simulink library "lib_all"  (Simulink 7.6)
settings: Solver=ode45  SolverMode=SingleTasking  StartTime=0.0  StopTime=10.0  RelTol=1e-3
values from neighbouring files (symbols this model uses):
  map_lib_03 = [6.8679 7.0159 7.4571 8.1849 9.1892 10.456 11.97 13.71 15.655 17.781 20.063 22.474 ... (803 elements, 73x11)]    [scr_simdata.mat]    (also in scr_simdata_all.mat)
  map_lib_06 = [2.5784 2.6727 2.9588 3.4453 4.1444 5.068 6.226 7.6242 9.2634 11.139 13.24 15.552 ... (803 elements, 73x11)]    [scr_simdata.mat]    (also in scr_simdata_all.mat)
  map_lib_09 = [0.0025784 0.0026727 0.0029588 0.0034453 0.0041444 0.005068 0.006226 0.0076242 0.0092634 0.011139 0.01324 0.015552 ... (803 elements, 73x11)]    [scr_simdata.mat]    (also in scr_simdata_all.mat)
  map_lib_10 = [2.74 2.75 2.76 2.77 2.78 2.79 2.8 2.81 2.82 2.83 2.84 2.85 ... (81 elements, 1x81)]    [scr_simdata.mat]    (also in scr_simdata_all.mat)
  map_lib_11 = [1.1983 1.2027 1.207 1.2113 1.2156 1.2198 1.2241 1.2283 1.2326 1.2368 1.241 1.2451 ... (81 elements, 1x81)]    [scr_simdata.mat]    (also in scr_simdata_all.mat)
  map_lib_12 = [2.74 2.75 2.76 2.77 2.78 2.79 2.8 2.81 2.82 2.83 2.84 2.85 ... (81 elements, 1x81)]    [scr_simdata.mat]    (also in scr_simdata_all.mat)
  map_lib_13 = [1.74 1.75 1.76 1.77 1.78 1.79 1.8 1.81 1.82 1.83 1.84 1.85 ... (81 elements, 1x81)]    [scr_simdata.mat]    (also in scr_simdata_all.mat)
  map_lib_16 = [0.00048828125 1.6159 1.8962 2.0821 2.2251 2.3426 2.4433 2.5318 2.611 2.683 2.749 2.8101 ... (1419 elements, 129x11)]    [scr_simdata.mat]    (also in scr_simdata_all.mat)
  map_mr2_01 = [0 800 1600 2400 3200 4000]    [scr_simdata.mat]    (also in scr_simdata_all.mat)
  map_mr2_02 = [1.32 1.32 1.33 1.34 1.36 1.38]    [scr_simdata.mat]    (also in scr_simdata_all.mat)
  map_mr2_03 = [0 800 1600 2400 3200 4000]    [scr_simdata.mat]    (also in scr_simdata_all.mat)
  map_mr2_04 = [1.32 1.32 1.33 1.34 1.36 1.38]    [scr_simdata.mat]    (also in scr_simdata_all.mat)
  map_mr2_05 = [0 800 1600 2400 3200 4000]    [scr_simdata.mat]    (also in scr_simdata_all.mat)
  map_mr2_06 = [1.32 1.32 1.33 1.34 1.36 1.38]    [scr_simdata.mat]    (also in scr_simdata_all.mat)
  Parameter1 = 6    [scr_simdata.mat]    (also in scr_simdata_all.mat)
  Parameter101 = 30    [scr_simdata.mat]    (also in scr_simdata_all.mat)
  Parameter102 = 12    [scr_simdata.mat]    (also in scr_simdata_all.mat)
  Parameter103 = -240    [scr_simdata.mat]    (also in scr_simdata_all.mat)
  Parameter108 = 48    [scr_simdata.mat]    (also in scr_simdata_all.mat)
  Parameter120 = 16    [scr_simdata.mat]    (also in scr_simdata_all.mat)
  Parameter121 = 87    [scr_simdata.mat]    (also in scr_simdata_all.mat)
  Parameter123 = 78    [scr_simdata.mat]    (also in scr_simdata_all.mat)
  Parameter124 = 60    [scr_simdata.mat]    (also in scr_simdata_all.mat)
  Parameter125 = -480    [scr_simdata.mat]    (also in scr_simdata_all.mat)
  Parameter130 = 96    [scr_simdata.mat]    (also in scr_simdata_all.mat)
  Parameter131 = 3    [scr_simdata.mat]    (also in scr_simdata_all.mat)
  Parameter144 = 3    [scr_simdata.mat]    (also in scr_simdata_all.mat)
  Parameter18 = 128    [scr_simdata.mat]    (also in scr_simdata_all.mat)
  Parameter19 = 16    [scr_simdata.mat]    (also in scr_simdata_all.mat)
  Parameter2 = 12    [scr_simdata.mat]    (also in scr_simdata_all.mat)
  Parameter20 = 7    [scr_simdata.mat]    (also in scr_simdata_all.mat)
  Parameter21 = 4    [scr_simdata.mat]    (also in scr_simdata_all.mat)
  Parameter3 = 18    [scr_simdata.mat]    (also in scr_simdata_all.mat)
  Parameter32 = 1    [scr_simdata.mat]    (also in scr_simdata_all.mat)
  Parameter34 = 24    [scr_simdata.mat]    (also in scr_simdata_all.mat)
  Parameter4 = 138    [scr_simdata.mat]    (also in scr_simdata_all.mat)
  Parameter42 = 48    [scr_simdata.mat]    (also in scr_simdata_all.mat)
  Parameter5 = 135    [scr_simdata.mat]    (also in scr_simdata_all.mat)
  Parameter55 = 0    [scr_simdata.mat]    (also in scr_simdata_all.mat)
  Parameter56 = 0    [scr_simdata.mat]    (also in scr_simdata_all.mat)
  Parameter57 = 1    [scr_simdata.mat]    (also in scr_simdata_all.mat)
  Parameter58 = 1    [scr_simdata.mat]    (also in scr_simdata_all.mat)
  Parameter59 = 2    [scr_simdata.mat]    (also in scr_simdata_all.mat)
  Parameter6 = 132    [scr_simdata.mat]    (also in scr_simdata_all.mat)
  Parameter60 = 2    [scr_simdata.mat]    (also in scr_simdata_all.mat)
  Parameter63 = 144    [scr_simdata.mat]    (also in scr_simdata_all.mat)
  Parameter7 = 126    [scr_simdata.mat]    (also in scr_simdata_all.mat)
  Parameter76 = 64    [scr_simdata.mat]    (also in scr_simdata_all.mat)
  Parameter77 = 135    [scr_simdata.mat]    (also in scr_simdata_all.mat)
  Parameter79 = 126    [scr_simdata.mat]    (also in scr_simdata_all.mat)
  Parameter80 = 108    [scr_simdata.mat]    (also in scr_simdata_all.mat)
  Parameter81 = 0    [scr_simdata.mat]    (also in scr_simdata_all.mat)
  Parameter86 = 0    [scr_simdata.mat]    (also in scr_simdata_all.mat)
  Parameter98 = 112    [scr_simdata.mat]    (also in scr_simdata_all.mat)
  Parameter99 = 39    [scr_simdata.mat]    (also in scr_simdata_all.mat)
undefined symbols (not defined in the file or in its folder): cylvolkp_hih_map2h, cylvolkp_map2h, pk1k_map2h
libraries: simulink x2
(part 10 of 36)

== system lib_all/SubSystem800/SubSystem955/SubSystem958 ==
  note: ***編集禁止***
  input : Inport
  element : Inport  Port=2
  Assignment : Assignment  IndexOptions=Index vector (port)  Indices=1  OutputSizes=[1 1]
  Inside_DSR : DataStoreRead  DataStoreName=DSM183
  Inside_DSW : DataStoreWrite  DataStoreName=DSM183
  wires:
  element/1 -> Assignment/3
  input/1 -> Assignment/2
  Inside_DSR/1 -> Assignment/1
  Assignment/1 -> Inside_DSW/1

== system lib_all/SubSystem959/SubSystem963/SubSystem1018 ==
  Block2612 : Inport
  Block2613 : Inport  Port=2
  Block2614 : Inport  Port=3
  Block2615 : Sum  OutDataTypeStr=double  RndMeth=Zero  SaturateOnIntegerOverflow=on  InputSameDT=on
  Block2616 : Sum  Inputs=+-  OutDataTypeStr=double  RndMeth=Zero  SaturateOnIntegerOverflow=on  InputSameDT=on
  Block2617 : Switch  Threshold=0.5  RndMeth=Zero  SaturateOnIntegerOverflow=on  InputSameDT=on
  Block2618 : Switch  Threshold=0.5  RndMeth=Zero  SaturateOnIntegerOverflow=on  InputSameDT=on
  SubSystem1019 : Subsystem
  SubSystem1020 : Subsystem
  Block2619 : Outport
  wires:
  Block2613/1 -> SubSystem1019/1(In1), Block2616/1, SubSystem1020/1(In1), Block2618/3, Block2615/2
  Block2612/1 -> Block2615/1, Block2616/2, SubSystem1019/2(In2)
  SubSystem1019/1(Out1) -> Block2617/2
  Block2614/1 -> SubSystem1020/2(In2)
  SubSystem1020/1(Out1) -> Block2618/2
  Block2618/1 -> Block2617/3
  Block2615/1 -> Block2618/1
  Block2617/1 -> Block2619/1
  Block2616/1 -> Block2617/1

== system lib_all/SubSystem959/SubSystem963/SubSystem1021 ==
  note: ***編集禁止***
  element : Inport
  Inside_DSR : DataStoreRead  DataStoreName=DSM49
  Selector : Selector  IndexOptions=Index vector (port)  Indices=[1 3]  OutputSizes=1
  Output : Outport
  wires:
  Selector/1 -> Output/1
  element/1 -> Selector/2
  Inside_DSR/1 -> Selector/1

== system lib_all/SubSystem959/SubSystem963/SubSystem1022 ==
  note: ***編集禁止***
  element : Inport
  Inside_DSR : DataStoreRead  DataStoreName=DSM36
  Selector : Selector  IndexOptions=Index vector (port)  Indices=[1 3]  OutputSizes=1
  Output : Outport
  wires:
  Selector/1 -> Output/1
  element/1 -> Selector/2
  Inside_DSR/1 -> Selector/1

== system lib_all/SubSystem959/SubSystem963/SubSystem964 ==
  note: ***編集禁止***
  In1 : Inport
  In2 : Inport  Port=2
  RO1 : RelationalOperator  Operator===  InputSameDT=on
  single1 : DataTypeConversion  OutDataTypeStr=single  SaturateOnIntegerOverflow=on  RndMeth=Zero
  single2 : DataTypeConversion  OutDataTypeStr=single  SaturateOnIntegerOverflow=on  RndMeth=Zero
  Out1 : Outport
  wires:
  RO1/1 -> Out1/1
  In2/1 -> single2/1
  In1/1 -> single1/1
  single1/1 -> RO1/1
  single2/1 -> RO1/2

== system lib_all/SubSystem959/SubSystem963/SubSystem965 ==
  note: Text185
  Block2490 : Inport
  Block2491 : Inport  Port=2
  Block2492 : Inport  Port=3
  Block2493 : Inport  Port=4
  Block2494 : Constant  Value=0  OutDataTypeStr=double  SampleTime=-1
  Block2495 : Constant  OutDataTypeStr=double  SampleTime=-1
  Block2496 : Constant  Value=0  OutDataTypeStr=double  SampleTime=-1
  Block2497 : Gain  Gain=1/5  SaturateOnIntegerOverflow=on  OutDataTypeStr=Inherit: Same as input  ParamDataTypeStr=Inherit: Same as input
      settings: AttributesFormatString=Attribute508
  Block2498 : Product  OutDataTypeStr=double  SaturateOnIntegerOverflow=on  RndMeth=Zero  InputSameDT=on
  Block2499 : Sum  Inputs=-+  OutDataTypeStr=double  RndMeth=Zero  SaturateOnIntegerOverflow=on  InputSameDT=on
  Block2500 : Sum  OutDataTypeStr=double  RndMeth=Zero  SaturateOnIntegerOverflow=on  InputSameDT=on
  Block2501 : Sum  OutDataTypeStr=double  RndMeth=Zero  SaturateOnIntegerOverflow=on  InputSameDT=on
  Block2502 : Switch  Threshold=0.5  RndMeth=Zero  SaturateOnIntegerOverflow=on  InputSameDT=on
  SubSystem967 : Subsystem
  SubSystem968 : Subsystem
  SubSystem974 : Subsystem
  Block2611 : Outport
  wires:
  Block2491/1 -> SubSystem968/1(Block2503)
  SubSystem968/1(Block2528) -> SubSystem974/1(Block2530)
  Block2493/1 -> SubSystem974/2(Block2531)
  SubSystem974/1(Block2609) -> Block2500/1, Block2499/1
  SubSystem974/2(Block2610) -> Block2499/2
  Block2499/1 -> Block2498/1  "SigLabel85"
  SubSystem968/2(Block2529) -> Block2498/2
  Block2500/1 -> Block2502/3, SubSystem967/1(In1)
  Block2492/1 -> SubSystem968/3(Block2505)
  Block2496/1 -> SubSystem967/2(In2)
  SubSystem967/1(Out1) -> Block2502/2
  Block2502/1 -> Block2611/1
  Block2494/1 -> Block2502/1
  Block2495/1 -> Block2501/2
  Block2498/1 -> Block2497/1
  Block2497/1 -> Block2500/2
  Block2490/1 -> Block2501/1
  Block2501/1 -> SubSystem968/2(Block2504), SubSystem974/3(Block2532)

== system lib_all/SubSystem1023/SubSystem1025/SubSystem1038/SubSystem1040 ==
  note: ***編集禁止***
  In1 : Inport
  In2 : Inport  Port=2
  RO1 : RelationalOperator  Operator===  InputSameDT=on
  single1 : DataTypeConversion  OutDataTypeStr=single  SaturateOnIntegerOverflow=on  RndMeth=Zero
  single2 : DataTypeConversion  OutDataTypeStr=single  SaturateOnIntegerOverflow=on  RndMeth=Zero
  Out1 : Outport
  wires:
  single2/1 -> RO1/2
  single1/1 -> RO1/1
  In1/1 -> single1/1
  In2/1 -> single2/1
  RO1/1 -> Out1/1

== system lib_all/SubSystem1023/SubSystem1025/SubSystem1038/SubSystem1041 ==
  note: Text189
  Block2707 : Inport
  Block2708 : Inport  Port=2
  Block2709 : Constant  Value=0  OutDataTypeStr=double  SampleTime=-1
  Block2710 : Constant  Value=720  OutDataTypeStr=double  SampleTime=-1
  Block2711 : Gain  Gain=5  SaturateOnIntegerOverflow=on  OutDataTypeStr=Inherit: Same as input  ParamDataTypeStr=Inherit: Same as input
      settings: AttributesFormatString=Attribute537
  SubSystem1042 : Subsystem
      settings: RTWFcnNameOpts=Use subsystem name
  SubSystem1047 : Subsystem
      settings: RTWSystemCode=Inline
  Block2737 : Outport
  wires:
  Block2709/1 -> SubSystem1047/3(Block2733)
  Block2710/1 -> SubSystem1047/1(Block2731)
  SubSystem1047/1(Block2736) -> Block2737/1
  Block2707/1 -> SubSystem1042/1(Block2712)
  Block2708/1 -> SubSystem1042/2(Block2713)
  SubSystem1042/1(Block2730) -> Block2711/1
  Block2711/1 -> SubSystem1047/2(Block2732)

== system lib_all/SubSystem1023/SubSystem1025/SubSystem1038/SubSystem1049 ==
  Block2738 : Inport
  Block2739 : Inport  Port=2
  Block2740 : Inport  Port=3
  Block2741 : EnablePort
  Block2742 : DataStoreWrite  DataStoreName=DSM12
  Block2743 : DataStoreWrite  DataStoreName=DSM14
  Block2744 : DataStoreWrite  DataStoreName=DSM13
  wires:
  Block2739/1 -> Block2743/1
  Block2740/1 -> Block2744/1
  Block2738/1 -> Block2742/1

== system lib_all/SubSystem1023/SubSystem1025/SubSystem1038/SubSystem1050 ==
  note: Text190
  Block2745 : Inport
  Block2746 : Inport  Port=2
  Block2747 : Constant  Value=360  OutDataTypeStr=double  SampleTime=-1
  Block2748 : Constant  Value=360  OutDataTypeStr=double  SampleTime=-1
  Block2749 : Sum  Inputs=+-  OutDataTypeStr=double  RndMeth=Zero  SaturateOnIntegerOverflow=on  InputSameDT=on
  Block2750 : Switch  Threshold=0.5  RndMeth=Zero  SaturateOnIntegerOverflow=on  InputSameDT=on
  Block2751 : Lookup2D  RowIndex=map_lib_05  ColumnIndex=map_lib_04  Table=map_lib_06  SaturateOnIntegerOverflow=on  InputSameDT=on
      settings: AttributesFormatString=<%<OutputValues>>\nAttribute546
  SubSystem1051 : Subsystem
  Block2752 : Outport
  wires:
  Block2751/1 -> Block2752/1
  Block2747/1 -> Block2749/2
  Block2749/1 -> Block2750/1
  SubSystem1051/1(Out1) -> Block2750/2
  Block2748/1 -> SubSystem1051/2(In2)
  Block2746/1 -> Block2749/1, Block2750/3, SubSystem1051/1(In1)
  Block2745/1 -> Block2751/2
  Block2750/1 -> Block2751/1  "SigLabel86"

== system lib_all/SubSystem1023/SubSystem1025/SubSystem1052/SubSystem1054 ==
  note: Text194
  note: Text193
  Block2763 : Inport
  Block2764 : Inport  Port=2
  Block2765 : Constant  Value=0  OutDataTypeStr=double  SampleTime=-1
  Block2766 : Constant  Value=0  OutDataTypeStr=double  SampleTime=-1
  Block2767 : Constant  Value=720  OutDataTypeStr=double  SampleTime=-1
  Block2768 : Constant  Value=5  OutDataTypeStr=double  SampleTime=-1
  Block2769 : DataStoreRead  DataStoreName=DSM50
  Block2770 : DataStoreRead  DataStoreName=DSM35
  Block2771 : Gain  Gain=5  SaturateOnIntegerOverflow=on  OutDataTypeStr=Inherit: Same as input  ParamDataTypeStr=Inherit: Same as input
      settings: AttributesFormatString=Attribute554
  Block2772 : Gain  Gain=5  SaturateOnIntegerOverflow=on  OutDataTypeStr=Inherit: Same as input  ParamDataTypeStr=Inherit: Same as input
      settings: AttributesFormatString=Attribute555
  Block2773 : Gain  Gain=1/5  SaturateOnIntegerOverflow=on  OutDataTypeStr=Inherit: Same as input  ParamDataTypeStr=Inherit: Same as input
      settings: AttributesFormatString=Attribute556
  Block2774 : Product  Inputs=*/  OutDataTypeStr=double  SaturateOnIntegerOverflow=on  RndMeth=Zero  InputSameDT=on
  Block2775 : Rounding
  Block2776 : Rounding
  Block2777 : Sum  Inputs=+-+  OutDataTypeStr=double  RndMeth=Zero  SaturateOnIntegerOverflow=on  InputSameDT=on
  Block2778 : Sum  Inputs=-+  OutDataTypeStr=double  RndMeth=Zero  SaturateOnIntegerOverflow=on  InputSameDT=on
  SubSystem1055 : Subsystem  TreatAsAtomicUnit=on  mask(***ATC.DIVISION***){bd_version=1.01.A, r0=チェック有り, r1=, r2=, r3=, r4=, r5=, r6=, r7=, r8=, r9=, c0=, c1=, c2=, c3=, c4=, c5=, c6=, c7=, c8=, c9=}
      mask prompts: bd_version "Version"
      mask help: これはAutoCからのブロックです．編集禁止．
      settings: OpenFcn=warndlg('変更禁止!');
      mask Help: web(ui_blockhelpmng(gcb));
  SubSystem1056 : Subsystem  TreatAsAtomicUnit=on  mask(***ATC.DSRVEC***){bd_version=1.01.A, r0=DSM95, r1=-1, r2=, r3=, r4=, r5=, r6=, r7=, r8=, r9=, c0=, c1=, c2=, c3=, c4=, c5=, c6=, c7=, c8=, c9=}
      mask prompts: bd_version "Version"
      mask help: これはAutoCからのブロックです．編集禁止．
      settings: OpenFcn=warndlg('変更禁止!');
      mask Help: web(ui_blockhelpmng(gcb));
      mask Display: disp('ATC.DSRVEC')
  SubSystem1057 : Subsystem
      settings: RTWSystemCode=Inline
  Block2787 : Outport
  Block2788 : Outport  Port=2
  wires:
  SubSystem1055/1(Out) -> Block2777/1  "SigLabel88"
  Block2763/1 -> Block2772/1
  Block2771/1 -> Block2778/1
  Block2775/1 -> Block2771/1
  Block2774/1 -> Block2775/1
  SubSystem1057/1(Block2786) -> Block2778/2, Block2773/1, Block2774/1
  Block2766/1 -> SubSystem1057/3(Block2781)
  Block2777/1 -> SubSystem1057/2(Block2780)  "SigLabel87"
  Block2767/1 -> SubSystem1057/1(Block2779)
  Block2764/1 -> SubSystem1056/1(element)
  Block2778/1 -> Block2788/1
  Block2768/1 -> Block2774/2
  Block2765/1 -> SubSystem1055/3(init)
  SubSystem1056/1(Output) -> SubSystem1055/1(mult)
  Block2770/1 -> SubSystem1055/2(div)
  Block2769/1 -> Block2777/2
  Block2772/1 -> Block2777/3
  Block2773/1 -> Block2776/1
  Block2776/1 -> Block2787/1

== system lib_all/SubSystem1023/SubSystem1025/SubSystem1052/SubSystem1060 ==
  note: Text197
  note: Text196
  note: Text195
  Block2789 : Inport
  Block2790 : Inport  Port=2
  Block2791 : Inport  Port=3
  Block2792 : Constant  Value=0  OutDataTypeStr=double  SampleTime=-1
  Block2793 : Constant  OutDataTypeStr=double  SampleTime=-1
  Block2794 : Constant  OutDataTypeStr=double  SampleTime=-1
  Block2795 : Constant  Value=0  OutDataTypeStr=double  SampleTime=-1
  Block2796 : Constant  OutDataTypeStr=double  SampleTime=-1
  Block2797 : Constant  Value=143  OutDataTypeStr=double  SampleTime=-1
  Block2798 : Demux  Outputs=2
  Block2799 : Logic  Operator=NOT
  Block2800 : Mux  Inputs=2
  Block2801 : Mux  Inputs=2
  Block2802 : Sum  OutDataTypeStr=double  RndMeth=Zero  SaturateOnIntegerOverflow=on  InputSameDT=on
  Block2803 : Sum  OutDataTypeStr=double  RndMeth=Zero  SaturateOnIntegerOverflow=on  InputSameDT=on
  Block2804 : Switch  Threshold=0.5  RndMeth=Zero  SaturateOnIntegerOverflow=on  InputSameDT=on
  Block2805 : Switch  Threshold=0.5  RndMeth=Zero  SaturateOnIntegerOverflow=on  InputSameDT=on
  Block2806 : Switch  Threshold=0.5  RndMeth=Zero  SaturateOnIntegerOverflow=on  InputSameDT=on
  SubSystem1061 : Subsystem
  SubSystem1062 : Subsystem
  SubSystem1063 : Subsystem
  SubSystem1064 : Subsystem  TreatAsAtomicUnit=on
  SubSystem1073 : Subsystem  TreatAsAtomicUnit=on
  SubSystem1083 : Subsystem  TreatAsAtomicUnit=on
  Block2851 : Outport
  Block2852 : Outport  Port=2
  wires:
  Block2803/1 -> SubSystem1083/1(Block2836), SubSystem1073/1(Block2821), SubSystem1064/1(Block2807)
  Block2796/1 -> Block2803/1
  Block2806/1 -> Block2803/2
  Block2791/1 -> SubSystem1083/2(Block2837), SubSystem1073/2(Block2822), SubSystem1064/2(Block2808)
  Block2800/1 -> Block2806/1
  Block2802/1 -> Block2800/2
  Block2801/1 -> Block2806/3
  Block2795/1 -> Block2801/2
  Block2794/1 -> Block2802/1
  SubSystem1061/1(Out1) -> Block2806/2
  Block2804/1 -> Block2798/1
  Block2798/2 -> Block2852/1
  Block2798/1 -> Block2851/1
  SubSystem1083/1(Block2850) -> Block2805/3
  Block2805/1 -> Block2804/3
  Block2799/1 -> SubSystem1083/Enable
  SubSystem1073/1(Block2835) -> Block2805/1
  SubSystem1063/1(Out1) -> Block2799/1, SubSystem1073/Enable, Block2805/2
  Block2793/1 -> SubSystem1063/2(In2)
  SubSystem1064/1(Block2820) -> Block2804/1
  SubSystem1062/1(Out1) -> SubSystem1064/Enable, Block2804/2
  Block2792/1 -> SubSystem1062/2(In2)
  Block2790/1 -> SubSystem1063/1(In1), SubSystem1062/1(In1)
  Block2797/1 -> Block2801/1, SubSystem1061/2(In2)
  Block2789/1 -> Block2800/1, Block2802/2, SubSystem1061/1(In1)

== system lib_all/SubSystem1023/SubSystem1093/SubSystem1106/SubSystem1108 ==
  note: ***編集禁止***
  In1 : Inport
  In2 : Inport  Port=2
  RO1 : RelationalOperator  Operator===  InputSameDT=on
  single1 : DataTypeConversion  OutDataTypeStr=single  SaturateOnIntegerOverflow=on  RndMeth=Zero
  single2 : DataTypeConversion  OutDataTypeStr=single  SaturateOnIntegerOverflow=on  RndMeth=Zero
  Out1 : Outport
  wires:
  single2/1 -> RO1/2
  single1/1 -> RO1/1
  In1/1 -> single1/1
  In2/1 -> single2/1
  RO1/1 -> Out1/1

== system lib_all/SubSystem1023/SubSystem1093/SubSystem1106/SubSystem1109 ==
  note: Text208
  Block2932 : Inport
  Block2933 : Inport  Port=2
  Block2934 : Constant  Value=0  OutDataTypeStr=double  SampleTime=-1
  Block2935 : Constant  Value=720  OutDataTypeStr=double  SampleTime=-1
  Block2936 : Gain  Gain=5  SaturateOnIntegerOverflow=on  OutDataTypeStr=Inherit: Same as input  ParamDataTypeStr=Inherit: Same as input
      settings: AttributesFormatString=Attribute574
  SubSystem1110 : Subsystem
      settings: RTWFcnNameOpts=Use subsystem name
  SubSystem1115 : Subsystem
      settings: RTWSystemCode=Inline
  Block2962 : Outport
  wires:
  Block2934/1 -> SubSystem1115/3(Block2958)
  Block2935/1 -> SubSystem1115/1(Block2956)
  SubSystem1115/1(Block2961) -> Block2962/1
  Block2932/1 -> SubSystem1110/1(Block2937)
  Block2933/1 -> SubSystem1110/2(Block2938)
  SubSystem1110/1(Block2955) -> Block2936/1
  Block2936/1 -> SubSystem1115/2(Block2957)

== system lib_all/SubSystem1023/SubSystem1093/SubSystem1106/SubSystem1117 ==
  Block2963 : Inport
  Block2964 : Inport  Port=2
  Block2965 : Inport  Port=3
  Block2966 : EnablePort
  Block2967 : DataStoreWrite  DataStoreName=DSM12
  Block2968 : DataStoreWrite  DataStoreName=DSM14
  Block2969 : DataStoreWrite  DataStoreName=DSM13
  wires:
  Block2964/1 -> Block2968/1
  Block2965/1 -> Block2969/1
  Block2963/1 -> Block2967/1

== system lib_all/SubSystem1023/SubSystem1093/SubSystem1106/SubSystem1118 ==
  note: Text209
  Block2970 : Inport
  Block2971 : Inport  Port=2
  Block2972 : Constant  Value=360  OutDataTypeStr=double  SampleTime=-1
  Block2973 : Constant  Value=360  OutDataTypeStr=double  SampleTime=-1
  Block2974 : Sum  Inputs=+-  OutDataTypeStr=double  RndMeth=Zero  SaturateOnIntegerOverflow=on  InputSameDT=on
  Block2975 : Switch  Threshold=0.5  RndMeth=Zero  SaturateOnIntegerOverflow=on  InputSameDT=on
  Block2976 : Lookup2D  RowIndex=map_lib_05  ColumnIndex=map_lib_04  Table=map_lib_06  SaturateOnIntegerOverflow=on  InputSameDT=on
      settings: AttributesFormatString=<%<OutputValues>>\nAttribute583
  SubSystem1119 : Subsystem
  Block2977 : Outport
  wires:
  Block2976/1 -> Block2977/1
  Block2972/1 -> Block2974/2
  Block2974/1 -> Block2975/1
  SubSystem1119/1(Out1) -> Block2975/2
  Block2973/1 -> SubSystem1119/2(In2)
  Block2971/1 -> Block2974/1, Block2975/3, SubSystem1119/1(In1)
  Block2970/1 -> Block2976/2
  Block2975/1 -> Block2976/1  "SigLabel96"

== system lib_all/SubSystem1023/SubSystem1093/SubSystem1120/SubSystem1122 ==
  note: Text213
  note: Text212
  Block2988 : Inport
  Block2989 : Inport  Port=2
  Block2990 : Constant  Value=0  OutDataTypeStr=double  SampleTime=-1
  Block2991 : Constant  Value=0  OutDataTypeStr=double  SampleTime=-1
  Block2992 : Constant  Value=720  OutDataTypeStr=double  SampleTime=-1
  Block2993 : Constant  Value=5  OutDataTypeStr=double  SampleTime=-1
  Block2994 : DataStoreRead  DataStoreName=DSM50
  Block2995 : DataStoreRead  DataStoreName=DSM35
  Block2996 : Gain  Gain=5  SaturateOnIntegerOverflow=on  OutDataTypeStr=Inherit: Same as input  ParamDataTypeStr=Inherit: Same as input
      settings: AttributesFormatString=Attribute591
  Block2997 : Gain  Gain=5  SaturateOnIntegerOverflow=on  OutDataTypeStr=Inherit: Same as input  ParamDataTypeStr=Inherit: Same as input
      settings: AttributesFormatString=Attribute592
  Block2998 : Gain  Gain=1/5  SaturateOnIntegerOverflow=on  OutDataTypeStr=Inherit: Same as input  ParamDataTypeStr=Inherit: Same as input
      settings: AttributesFormatString=Attribute593
  Block2999 : Product  Inputs=*/  OutDataTypeStr=double  SaturateOnIntegerOverflow=on  RndMeth=Zero  InputSameDT=on
  Block3000 : Rounding
  Block3001 : Rounding
  Block3002 : Sum  Inputs=+-+  OutDataTypeStr=double  RndMeth=Zero  SaturateOnIntegerOverflow=on  InputSameDT=on
  Block3003 : Sum  Inputs=-+  OutDataTypeStr=double  RndMeth=Zero  SaturateOnIntegerOverflow=on  InputSameDT=on
  SubSystem1123 : Subsystem  TreatAsAtomicUnit=on  mask(***ATC.DIVISION***){bd_version=1.01.A, r0=チェック有り, r1=, r2=, r3=, r4=, r5=, r6=, r7=, r8=, r9=, c0=, c1=, c2=, c3=, c4=, c5=, c6=, c7=, c8=, c9=}
      mask prompts: bd_version "Version"
      mask help: これはAutoCからのブロックです．編集禁止．
      settings: OpenFcn=warndlg('変更禁止!');
      mask Help: web(ui_blockhelpmng(gcb));
  SubSystem1124 : Subsystem  TreatAsAtomicUnit=on  mask(***ATC.DSRVEC***){bd_version=1.01.A, r0=DSM95, r1=-1, r2=, r3=, r4=, r5=, r6=, r7=, r8=, r9=, c0=, c1=, c2=, c3=, c4=, c5=, c6=, c7=, c8=, c9=}
      mask prompts: bd_version "Version"
      mask help: これはAutoCからのブロックです．編集禁止．
      settings: OpenFcn=warndlg('変更禁止!');
      mask Help: web(ui_blockhelpmng(gcb));
      mask Display: disp('ATC.DSRVEC')
  SubSystem1125 : Subsystem
      settings: RTWSystemCode=Inline
  Block3012 : Outport
  Block3013 : Outport  Port=2
  wires:
  SubSystem1123/1(Out) -> Block3002/1  "SigLabel98"
  Block2988/1 -> Block2997/1
  Block2996/1 -> Block3003/1
  Block3000/1 -> Block2996/1
  Block2999/1 -> Block3000/1
  SubSystem1125/1(Block3011) -> Block3003/2, Block2998/1, Block2999/1
  Block2991/1 -> SubSystem1125/3(Block3006)
  Block3002/1 -> SubSystem1125/2(Block3005)  "SigLabel97"
  Block2992/1 -> SubSystem1125/1(Block3004)
  Block2989/1 -> SubSystem1124/1(element)
  Block3003/1 -> Block3013/1
  Block2993/1 -> Block2999/2
  Block2990/1 -> SubSystem1123/3(init)
  SubSystem1124/1(Output) -> SubSystem1123/1(mult)
  Block2995/1 -> SubSystem1123/2(div)
  Block2994/1 -> Block3002/2
  Block2997/1 -> Block3002/3
  Block2998/1 -> Block3001/1
  Block3001/1 -> Block3012/1

== system lib_all/SubSystem1023/SubSystem1093/SubSystem1120/SubSystem1128 ==
  note: Text216
  note: Text215
  note: Text214
  Block3014 : Inport
  Block3015 : Inport  Port=2
  Block3016 : Inport  Port=3
  Block3017 : Constant  Value=0  OutDataTypeStr=double  SampleTime=-1
  Block3018 : Constant  OutDataTypeStr=double  SampleTime=-1
  Block3019 : Constant  OutDataTypeStr=double  SampleTime=-1
  Block3020 : Constant  Value=0  OutDataTypeStr=double  SampleTime=-1
  Block3021 : Constant  OutDataTypeStr=double  SampleTime=-1
  Block3022 : Constant  Value=143  OutDataTypeStr=double  SampleTime=-1
  Block3023 : Demux  Outputs=2
  Block3024 : Logic  Operator=NOT
  Block3025 : Mux  Inputs=2
  Block3026 : Mux  Inputs=2
  Block3027 : Sum  OutDataTypeStr=double  RndMeth=Zero  SaturateOnIntegerOverflow=on  InputSameDT=on
  Block3028 : Sum  OutDataTypeStr=double  RndMeth=Zero  SaturateOnIntegerOverflow=on  InputSameDT=on
  Block3029 : Switch  Threshold=0.5  RndMeth=Zero  SaturateOnIntegerOverflow=on  InputSameDT=on
  Block3030 : Switch  Threshold=0.5  RndMeth=Zero  SaturateOnIntegerOverflow=on  InputSameDT=on
  Block3031 : Switch  Threshold=0.5  RndMeth=Zero  SaturateOnIntegerOverflow=on  InputSameDT=on
  SubSystem1129 : Subsystem
  SubSystem1130 : Subsystem
  SubSystem1131 : Subsystem
  SubSystem1132 : Subsystem  TreatAsAtomicUnit=on
  SubSystem1141 : Subsystem  TreatAsAtomicUnit=on
  SubSystem1151 : Subsystem  TreatAsAtomicUnit=on
  Block3076 : Outport
  Block3077 : Outport  Port=2
  wires:
  Block3028/1 -> SubSystem1151/1(Block3061), SubSystem1141/1(Block3046), SubSystem1132/1(Block3032)
  Block3021/1 -> Block3028/1
  Block3031/1 -> Block3028/2
  Block3016/1 -> SubSystem1151/2(Block3062), SubSystem1141/2(Block3047), SubSystem1132/2(Block3033)
  Block3025/1 -> Block3031/1
  Block3027/1 -> Block3025/2
  Block3026/1 -> Block3031/3
  Block3020/1 -> Block3026/2
  Block3019/1 -> Block3027/1
  SubSystem1129/1(Out1) -> Block3031/2
  Block3029/1 -> Block3023/1
  Block3023/2 -> Block3077/1
  Block3023/1 -> Block3076/1
  SubSystem1151/1(Block3075) -> Block3030/3
  Block3030/1 -> Block3029/3
  Block3024/1 -> SubSystem1151/Enable
  SubSystem1141/1(Block3060) -> Block3030/1
  SubSystem1131/1(Out1) -> Block3024/1, SubSystem1141/Enable, Block3030/2
  Block3018/1 -> SubSystem1131/2(In2)
  SubSystem1132/1(Block3045) -> Block3029/1
  SubSystem1130/1(Out1) -> SubSystem1132/Enable, Block3029/2
  Block3017/1 -> SubSystem1130/2(In2)
  Block3015/1 -> SubSystem1131/1(In1), SubSystem1130/1(In1)
  Block3022/1 -> Block3026/1, SubSystem1129/2(In2)
  Block3014/1 -> Block3025/1, Block3027/2, SubSystem1129/1(In1)

== system lib_all/SubSystem1023/SubSystem1161/SubSystem1164/SubSystem1165 ==
  Block3097 : Inport
  Block3098 : Inport  Port=2
  Block3099 : Constant  Value=Parameter32  OutDataTypeStr=double  SampleTime=-1
  Block3100 : Constant  Value=2  OutDataTypeStr=double  SampleTime=-1
  Block3101 : Constant  Value=Parameter42  OutDataTypeStr=double  SampleTime=-1
  Block3102 : Constant  Value=0  OutDataTypeStr=double  SampleTime=-1
  Block3103 : Constant  Value=72  OutDataTypeStr=double  SampleTime=-1
  Block3104 : Constant  Value=Parameter34  OutDataTypeStr=double  SampleTime=-1
  Block3105 : Logic  Operator=OR
  Block3106 : Sum  Inputs=+-  OutDataTypeStr=double  RndMeth=Zero  SaturateOnIntegerOverflow=on  InputSameDT=on
  Block3107 : Switch  Threshold=0.5  RndMeth=Zero  SaturateOnIntegerOverflow=on  InputSameDT=on
  Block3108 : Switch  Threshold=0.5  RndMeth=Zero  SaturateOnIntegerOverflow=on  InputSameDT=on
  SubSystem1166 : Subsystem
  SubSystem1167 : Subsystem
  SubSystem1168 : Subsystem
      settings: RTWSystemCode=Inline
  Block3115 : Outport
  wires:
  Block3108/1 -> Block3115/1
  Block3107/1 -> Block3106/2
  Block3104/1 -> Block3107/3
  Block3101/1 -> Block3107/1
  Block3105/1 -> Block3108/2
  SubSystem1167/1(Out1) -> Block3105/2
  SubSystem1166/1(Out1) -> Block3107/2, Block3105/1
  Block3100/1 -> SubSystem1167/2(In2)
  Block3099/1 -> SubSystem1166/1(In1)
  Block3098/1 -> SubSystem1167/1(In1), SubSystem1166/2(In2)
  Block3097/1 -> Block3106/1, Block3108/3
  Block3106/1 -> SubSystem1168/2(Block3110)
  Block3102/1 -> SubSystem1168/3(Block3111)
  Block3103/1 -> SubSystem1168/1(Block3109)
  SubSystem1168/1(Block3114) -> Block3108/1

== system lib_all/SubSystem1023/SubSystem1161/SubSystem1164/SubSystem1170 ==
  Block3116 : Inport
  Block3117 : Inport  Port=2
  Block3118 : Inport  Port=3
  Block3119 : Sum  OutDataTypeStr=double  RndMeth=Zero  SaturateOnIntegerOverflow=on  InputSameDT=on
  Block3120 : Switch  Threshold=0.5  RndMeth=Zero  SaturateOnIntegerOverflow=on  InputSameDT=on
  SubSystem1171 : Subsystem
  Block3121 : Outport
  wires:
  Block3116/1 -> Block3119/1
  Block3120/1 -> Block3121/1
  Block3119/1 -> Block3120/1
  SubSystem1171/1(Out1) -> Block3120/2
  Block3118/1 -> SubSystem1171/2(In2)
  Block3117/1 -> Block3119/2, Block3120/3, SubSystem1171/1(In1)

== system lib_all/SubSystem1023/SubSystem1161/SubSystem1173/SubSystem1174 ==
  note: ***編集禁止***
  In1 : Inport
  In2 : Inport  Port=2
  RO1 : RelationalOperator  Operator=>  InputSameDT=on
  single1 : DataTypeConversion  OutDataTypeStr=single  SaturateOnIntegerOverflow=on  RndMeth=Zero
  single2 : DataTypeConversion  OutDataTypeStr=single  SaturateOnIntegerOverflow=on  RndMeth=Zero
  Out1 : Outport
  wires:
  RO1/1 -> Out1/1
  In2/1 -> single2/1
  In1/1 -> single1/1
  single1/1 -> RO1/1
  single2/1 -> RO1/2

== system lib_all/SubSystem1023/SubSystem1175/SubSystem1178/SubSystem1179 ==
  Block3156 : Inport
  Block3157 : Inport  Port=2
  Block3158 : Constant  Value=Parameter32  OutDataTypeStr=double  SampleTime=-1
  Block3159 : Constant  Value=2  OutDataTypeStr=double  SampleTime=-1
  Block3160 : Constant  Value=Parameter42  OutDataTypeStr=double  SampleTime=-1
  Block3161 : Constant  Value=0  OutDataTypeStr=double  SampleTime=-1
  Block3162 : Constant  Value=72  OutDataTypeStr=double  SampleTime=-1
  Block3163 : Constant  Value=Parameter34  OutDataTypeStr=double  SampleTime=-1
  Block3164 : Logic  Operator=OR
  Block3165 : Sum  Inputs=+-  OutDataTypeStr=double  RndMeth=Zero  SaturateOnIntegerOverflow=on  InputSameDT=on
  Block3166 : Switch  Threshold=0.5  RndMeth=Zero  SaturateOnIntegerOverflow=on  InputSameDT=on
  Block3167 : Switch  Threshold=0.5  RndMeth=Zero  SaturateOnIntegerOverflow=on  InputSameDT=on
  SubSystem1180 : Subsystem
  SubSystem1181 : Subsystem
  SubSystem1182 : Subsystem
      settings: RTWSystemCode=Inline
  Block3174 : Outport
  wires:
  Block3167/1 -> Block3174/1
  Block3166/1 -> Block3165/2
  Block3163/1 -> Block3166/3
  Block3160/1 -> Block3166/1
  Block3164/1 -> Block3167/2
  SubSystem1181/1(Out1) -> Block3164/2
  SubSystem1180/1(Out1) -> Block3166/2, Block3164/1
  Block3159/1 -> SubSystem1181/2(In2)
  Block3158/1 -> SubSystem1180/1(In1)
  Block3157/1 -> SubSystem1181/1(In1), SubSystem1180/2(In2)
  Block3156/1 -> Block3165/1, Block3167/3
  Block3165/1 -> SubSystem1182/2(Block3169)
  Block3161/1 -> SubSystem1182/3(Block3170)
  Block3162/1 -> SubSystem1182/1(Block3168)
  SubSystem1182/1(Block3173) -> Block3167/1

== system lib_all/SubSystem1023/SubSystem1175/SubSystem1178/SubSystem1184 ==
  Block3175 : Inport
  Block3176 : Inport  Port=2
  Block3177 : Inport  Port=3
  Block3178 : Sum  OutDataTypeStr=double  RndMeth=Zero  SaturateOnIntegerOverflow=on  InputSameDT=on
  Block3179 : Switch  Threshold=0.5  RndMeth=Zero  SaturateOnIntegerOverflow=on  InputSameDT=on
  SubSystem1185 : Subsystem
  Block3180 : Outport
  wires:
  Block3175/1 -> Block3178/1
  Block3179/1 -> Block3180/1
  Block3178/1 -> Block3179/1
  SubSystem1185/1(Out1) -> Block3179/2
  Block3177/1 -> SubSystem1185/2(In2)
  Block3176/1 -> Block3178/2, Block3179/3, SubSystem1185/1(In1)

== system lib_all/SubSystem1023/SubSystem1175/SubSystem1187/SubSystem1188 ==
  note: ***編集禁止***
  In1 : Inport
  In2 : Inport  Port=2
  RO1 : RelationalOperator  Operator=>  InputSameDT=on
  single1 : DataTypeConversion  OutDataTypeStr=single  SaturateOnIntegerOverflow=on  RndMeth=Zero
  single2 : DataTypeConversion  OutDataTypeStr=single  SaturateOnIntegerOverflow=on  RndMeth=Zero
  Out1 : Outport
  wires:
  RO1/1 -> Out1/1
  In2/1 -> single2/1
  In1/1 -> single1/1
  single1/1 -> RO1/1
  single2/1 -> RO1/2

== system lib_all/SubSystem1196/SubSystem1206/SubSystem1208/SubSystem1210 ==
  note: ***編集禁止***
  In1 : Inport
  In2 : Inport  Port=2
  LO1 : Logic
  RO1 : RelationalOperator  Operator=<  InputSameDT=on
  RO2 : RelationalOperator  Operator=~=  InputSameDT=on
  single1 : DataTypeConversion  OutDataTypeStr=single  SaturateOnIntegerOverflow=on  RndMeth=Zero
  single2 : DataTypeConversion  OutDataTypeStr=single  SaturateOnIntegerOverflow=on  RndMeth=Zero
  Out1 : Outport
  wires:
  In2/1 -> single2/1
  In1/1 -> single1/1
  single1/1 -> RO1/1, RO2/1
  single2/1 -> RO1/2, RO2/2
  RO1/1 -> LO1/1
  RO2/1 -> LO1/2
  LO1/1 -> Out1/1

== system lib_all/SubSystem1196/SubSystem1206/SubSystem1208/SubSystem1211 ==
  note: Text238
  note: Text237
  Block3290 : Inport
  Block3291 : Inport  Port=2
  Block3292 : Inport  Port=3
  Block3293 : Constant  Value=720  OutDataTypeStr=double  SampleTime=-1
  Block3294 : Constant  Value=0  OutDataTypeStr=double  SampleTime=-1
  Block3295 : Constant  Value=0  OutDataTypeStr=double  SampleTime=-1
  Block3296 : Constant  Value=5  OutDataTypeStr=double  SampleTime=-1
  Block3297 : DataStoreRead  DataStoreName=DSM50
  Block3298 : DataStoreRead  DataStoreName=DSM35
  Block3299 : Gain  Gain=5  SaturateOnIntegerOverflow=on  OutDataTypeStr=Inherit: Same as input  ParamDataTypeStr=Inherit: Same as input
      settings: AttributesFormatString=Attribute657
  Block3300 : Gain  Gain=5  SaturateOnIntegerOverflow=on  OutDataTypeStr=Inherit: Same as input  ParamDataTypeStr=Inherit: Same as input
      settings: AttributesFormatString=Attribute658
  Block3301 : Gain  Gain=1/5  SaturateOnIntegerOverflow=on  OutDataTypeStr=Inherit: Same as input  ParamDataTypeStr=Inherit: Same as input
      settings: AttributesFormatString=Attribute659
  Block3302 : Product  Inputs=*/  OutDataTypeStr=double  SaturateOnIntegerOverflow=on  RndMeth=Zero  InputSameDT=on
  Block3303 : Rounding
  Block3304 : Rounding
  Block3305 : Sum  Inputs=+-+-  OutDataTypeStr=double  RndMeth=Zero  SaturateOnIntegerOverflow=on  InputSameDT=on
  Block3306 : Sum  Inputs=-+  OutDataTypeStr=double  RndMeth=Zero  SaturateOnIntegerOverflow=on  InputSameDT=on
  SubSystem1212 : Subsystem  TreatAsAtomicUnit=on  mask(***ATC.DIVISION***){bd_version=1.01.A, r0=チェック有り, r1=, r2=, r3=, r4=, r5=, r6=, r7=, r8=, r9=, c0=, c1=, c2=, c3=, c4=, c5=, c6=, c7=, c8=, c9=}
      mask prompts: bd_version "Version"
      mask help: これはAutoCからのブロックです．編集禁止．
      settings: OpenFcn=warndlg('変更禁止!');
      mask Help: web(ui_blockhelpmng(gcb));
  SubSystem1213 : Subsystem  TreatAsAtomicUnit=on  mask(***ATC.DSRVEC***){bd_version=1.01.A, r0=DSM95, r1=-1, r2=, r3=, r4=, r5=, r6=, r7=, r8=, r9=, c0=, c1=, c2=, c3=, c4=, c5=, c6=, c7=, c8=, c9=}
      mask prompts: bd_version "Version"
      mask help: これはAutoCからのブロックです．編集禁止．
      settings: OpenFcn=warndlg('変更禁止!');
      mask Help: web(ui_blockhelpmng(gcb));
      mask Display: disp('ATC.DSRVEC')
  SubSystem1214 : Subsystem
      settings: RTWSystemCode=Inline
  Block3315 : Outport
  Block3316 : Outport  Port=2
  wires:
  Block3304/1 -> Block3315/1
  Block3301/1 -> Block3304/1
  Block3300/1 -> Block3305/1
  Block3297/1 -> Block3305/2
  SubSystem1213/1(Output) -> SubSystem1212/1(mult)
  Block3294/1 -> SubSystem1212/3(init)
  SubSystem1212/1(Out) -> Block3305/3  "SigLabel109"
  Block3290/1 -> Block3300/1
  SubSystem1214/1(Block3314) -> Block3301/1, Block3306/2, Block3302/1
  Block3292/1 -> Block3305/4
  Block3293/1 -> SubSystem1214/1(Block3307)
  Block3305/1 -> SubSystem1214/2(Block3308)  "SigLabel108"
  Block3295/1 -> SubSystem1214/3(Block3309)
  Block3291/1 -> SubSystem1213/1(element)
  Block3296/1 -> Block3302/2
  Block3302/1 -> Block3303/1
  Block3303/1 -> Block3299/1
  Block3299/1 -> Block3306/1
  Block3306/1 -> Block3316/1
  Block3298/1 -> SubSystem1212/2(div)

== system lib_all/SubSystem1196/SubSystem1206/SubSystem1208/SubSystem1217 ==
  note: Text241
  note: Text240
  note: Text239
  Block3317 : Inport
  Block3318 : Inport  Port=2
  Block3319 : Inport  Port=3
  Block3320 : Constant  Value=0  OutDataTypeStr=double  SampleTime=-1
  Block3321 : Constant  OutDataTypeStr=double  SampleTime=-1
  Block3322 : Constant  OutDataTypeStr=double  SampleTime=-1
  Block3323 : Constant  Value=0  OutDataTypeStr=double  SampleTime=-1
  Block3324 : Constant  Value=2  OutDataTypeStr=double  SampleTime=-1
  Block3325 : Constant  OutDataTypeStr=double  SampleTime=-1
  Block3326 : Constant  Value=143  OutDataTypeStr=double  SampleTime=-1
  Block3327 : Demux  Outputs=2
  Block3328 : Logic  Operator=NOT
  Block3329 : Mux  Inputs=2
  Block3330 : Mux  Inputs=2
  Block3331 : Sum  OutDataTypeStr=double  RndMeth=Zero  SaturateOnIntegerOverflow=on  InputSameDT=on
  Block3332 : Sum  OutDataTypeStr=double  RndMeth=Zero  SaturateOnIntegerOverflow=on  InputSameDT=on
  Block{3333..3336} (4 alike) : Switch  Threshold=0.5  RndMeth=Zero  SaturateOnIntegerOverflow=on  InputSameDT=on
  SubSystem1218 : Subsystem
  SubSystem1219 : Subsystem
  SubSystem1220 : Subsystem
  SubSystem1221 : Subsystem
  SubSystem1222 : Subsystem  TreatAsAtomicUnit=on
  SubSystem1231 : Subsystem  TreatAsAtomicUnit=on
  SubSystem1241 : Subsystem  TreatAsAtomicUnit=on
  SubSystem1251 : Subsystem  TreatAsAtomicUnit=on
  Block3396 : Outport
  Block3397 : Outport  Port=2
  wires:
  Block3335/1 -> Block3332/2
  Block3325/1 -> Block3332/1
  Block3317/1 -> SubSystem1218/1(In1), Block3331/2, Block3329/1
  Block3326/1 -> SubSystem1218/2(In2), Block3330/1
  Block3318/1 -> SubSystem1219/1(In1), SubSystem1220/1(In1), SubSystem1221/1(In1)
  Block3320/1 -> SubSystem1219/2(In2)
  SubSystem1219/1(Out1) -> Block3333/2, SubSystem1222/Enable
  SubSystem1222/1(Block3350) -> Block3333/1
  Block3321/1 -> SubSystem1220/2(In2)
  SubSystem1220/1(Out1) -> Block3334/2, SubSystem1231/Enable
  SubSystem1231/1(Block3365) -> Block3334/1
  Block3334/1 -> Block3333/3
  Block3332/1 -> SubSystem1222/1(Block3337), SubSystem1231/1(Block3351), SubSystem1241/1(Block3366), SubSystem1251/1(Block3381)
  Block3327/1 -> Block3396/1
  Block3327/2 -> Block3397/1
  Block3333/1 -> Block3327/1
  SubSystem1218/1(Out1) -> Block3335/2
  Block3322/1 -> Block3331/1
  Block3323/1 -> Block3330/2
  Block3330/1 -> Block3335/3
  Block3331/1 -> Block3329/2
  Block3329/1 -> Block3335/1
  Block3324/1 -> SubSystem1221/2(In2)
  SubSystem1221/1(Out1) -> Block3328/1, SubSystem1241/Enable, Block3336/2
  Block3328/1 -> SubSystem1251/Enable
  SubSystem1241/1(Block3380) -> Block3336/1
  SubSystem1251/1(Block3395) -> Block3336/3
  Block3336/1 -> Block3334/3
  Block3319/1 -> SubSystem1222/2(Block3338), SubSystem1231/2(Block3352), SubSystem1241/2(Block3367), SubSystem1251/2(Block3382)

== system lib_all/SubSystem1196/SubSystem1206/SubSystem1261/SubSystem1263 ==
  note: Text243
  Block3403 : Inport
  Block3404 : Inport  Port=2
  Block3405 : Inport  Port=3
  Block3406 : Constant  Value=0  OutDataTypeStr=double  SampleTime=-1
  Block3407 : Constant  Value=720  OutDataTypeStr=double  SampleTime=-1
  Block3408 : Gain  Gain=5  SaturateOnIntegerOverflow=on  OutDataTypeStr=Inherit: Same as input  ParamDataTypeStr=Inherit: Same as input
      settings: AttributesFormatString=Attribute670
  Block3409 : Sum  Inputs=+-  OutDataTypeStr=double  RndMeth=Zero  SaturateOnIntegerOverflow=on  InputSameDT=on
  SubSystem1264 : Subsystem
      settings: RTWFcnNameOpts=Use subsystem name
  SubSystem1269 : Subsystem
      settings: RTWSystemCode=Inline
  Block3435 : Outport
  wires:
  Block3408/1 -> Block3409/1
  SubSystem1264/1(Block3428) -> Block3408/1
  Block3403/1 -> SubSystem1264/1(Block3410)
  Block3404/1 -> SubSystem1264/2(Block3411)
  Block3405/1 -> Block3409/2
  Block3409/1 -> SubSystem1269/2(Block3430)
  Block3407/1 -> SubSystem1269/1(Block3429)
  Block3406/1 -> SubSystem1269/3(Block3431)
  SubSystem1269/1(Block3434) -> Block3435/1

== system lib_all/SubSystem1196/SubSystem1206/SubSystem1261/SubSystem1271 ==
  note: Text244
  Block3436 : Inport
  Block3437 : Inport  Port=2
  Block3438 : Constant  Value=360  OutDataTypeStr=double  SampleTime=-1
  Block3439 : Constant  Value=360  OutDataTypeStr=double  SampleTime=-1
  Block3440 : Sum  Inputs=+-  OutDataTypeStr=double  RndMeth=Zero  SaturateOnIntegerOverflow=on  InputSameDT=on
  Block3441 : Switch  Threshold=0.5  RndMeth=Zero  SaturateOnIntegerOverflow=on  InputSameDT=on
  Block3442 : Lookup2D  RowIndex=map_lib_08  ColumnIndex=map_lib_07  Table=map_lib_09  SaturateOnIntegerOverflow=on  InputSameDT=on
      settings: AttributesFormatString=<%<OutputValues>>\nAttribute679
  SubSystem1272 : Subsystem
  Block3443 : Outport
  wires:
  Block3437/1 -> SubSystem1272/1(In1), Block3441/3, Block3440/1
  Block3439/1 -> SubSystem1272/2(In2)
  SubSystem1272/1(Out1) -> Block3441/2
  Block3440/1 -> Block3441/1
  Block3438/1 -> Block3440/2
  Block3442/1 -> Block3443/1
  Block3436/1 -> Block3442/2
  Block3441/1 -> Block3442/1  "SigLabel111"

== system lib_all/SubSystem1196/SubSystem1273/SubSystem1275/SubSystem1277 ==
  note: ***編集禁止***
  In1 : Inport
  In2 : Inport  Port=2
  LO1 : Logic
  RO1 : RelationalOperator  Operator=<  InputSameDT=on
  RO2 : RelationalOperator  Operator=~=  InputSameDT=on
  single1 : DataTypeConversion  OutDataTypeStr=single  SaturateOnIntegerOverflow=on  RndMeth=Zero
  single2 : DataTypeConversion  OutDataTypeStr=single  SaturateOnIntegerOverflow=on  RndMeth=Zero
  Out1 : Outport
  wires:
  In2/1 -> single2/1
  In1/1 -> single1/1
  single1/1 -> RO1/1, RO2/1
  single2/1 -> RO1/2, RO2/2
  RO1/1 -> LO1/1
  RO2/1 -> LO1/2
  LO1/1 -> Out1/1

== system lib_all/SubSystem1196/SubSystem1273/SubSystem1275/SubSystem1278 ==
  note: Text250
  note: Text249
  Block3465 : Inport
  Block3466 : Inport  Port=2
  Block3467 : Inport  Port=3
  Block3468 : Constant  Value=720  OutDataTypeStr=double  SampleTime=-1
  Block3469 : Constant  Value=0  OutDataTypeStr=double  SampleTime=-1
  Block3470 : Constant  Value=0  OutDataTypeStr=double  SampleTime=-1
  Block3471 : Constant  Value=5  OutDataTypeStr=double  SampleTime=-1
  Block3472 : DataStoreRead  DataStoreName=DSM50
  Block3473 : DataStoreRead  DataStoreName=DSM35
  Block3474 : Gain  Gain=5  SaturateOnIntegerOverflow=on  OutDataTypeStr=Inherit: Same as input  ParamDataTypeStr=Inherit: Same as input
      settings: AttributesFormatString=Attribute690
  Block3475 : Gain  Gain=5  SaturateOnIntegerOverflow=on  OutDataTypeStr=Inherit: Same as input  ParamDataTypeStr=Inherit: Same as input
      settings: AttributesFormatString=Attribute691
  Block3476 : Gain  Gain=1/5  SaturateOnIntegerOverflow=on  OutDataTypeStr=Inherit: Same as input  ParamDataTypeStr=Inherit: Same as input
      settings: AttributesFormatString=Attribute692
  Block3477 : Product  Inputs=*/  OutDataTypeStr=double  SaturateOnIntegerOverflow=on  RndMeth=Zero  InputSameDT=on
  Block3478 : Rounding
  Block3479 : Rounding
  Block3480 : Sum  Inputs=+-+-  OutDataTypeStr=double  RndMeth=Zero  SaturateOnIntegerOverflow=on  InputSameDT=on
  Block3481 : Sum  Inputs=-+  OutDataTypeStr=double  RndMeth=Zero  SaturateOnIntegerOverflow=on  InputSameDT=on
  SubSystem1279 : Subsystem  TreatAsAtomicUnit=on  mask(***ATC.DIVISION***){bd_version=1.01.A, r0=チェック有り, r1=, r2=, r3=, r4=, r5=, r6=, r7=, r8=, r9=, c0=, c1=, c2=, c3=, c4=, c5=, c6=, c7=, c8=, c9=}
      mask prompts: bd_version "Version"
      mask help: これはAutoCからのブロックです．編集禁止．
      settings: OpenFcn=warndlg('変更禁止!');
      mask Help: web(ui_blockhelpmng(gcb));
  SubSystem1280 : Subsystem  TreatAsAtomicUnit=on  mask(***ATC.DSRVEC***){bd_version=1.01.A, r0=DSM95, r1=-1, r2=, r3=, r4=, r5=, r6=, r7=, r8=, r9=, c0=, c1=, c2=, c3=, c4=, c5=, c6=, c7=, c8=, c9=}
      mask prompts: bd_version "Version"
      mask help: これはAutoCからのブロックです．編集禁止．
      settings: OpenFcn=warndlg('変更禁止!');
      mask Help: web(ui_blockhelpmng(gcb));
      mask Display: disp('ATC.DSRVEC')
  SubSystem1281 : Subsystem
      settings: RTWSystemCode=Inline
  Block3490 : Outport
  Block3491 : Outport  Port=2
  wires:
  Block3479/1 -> Block3490/1
  Block3476/1 -> Block3479/1
  Block3475/1 -> Block3480/1
  Block3472/1 -> Block3480/2
  SubSystem1280/1(Output) -> SubSystem1279/1(mult)
  Block3469/1 -> SubSystem1279/3(init)
  SubSystem1279/1(Out) -> Block3480/3  "SigLabel115"
  Block3465/1 -> Block3475/1
  SubSystem1281/1(Block3489) -> Block3476/1, Block3481/2, Block3477/1
  Block3467/1 -> Block3480/4
  Block3468/1 -> SubSystem1281/1(Block3482)
  Block3480/1 -> SubSystem1281/2(Block3483)  "SigLabel114"
  Block3470/1 -> SubSystem1281/3(Block3484)
  Block3466/1 -> SubSystem1280/1(element)
  Block3471/1 -> Block3477/2
  Block3477/1 -> Block3478/1
  Block3478/1 -> Block3474/1
  Block3474/1 -> Block3481/1
  Block3481/1 -> Block3491/1
  Block3473/1 -> SubSystem1279/2(div)

== system lib_all/SubSystem1196/SubSystem1273/SubSystem1275/SubSystem1284 ==
  note: Text253
  note: Text252
  note: Text251
  Block3492 : Inport
  Block3493 : Inport  Port=2
  Block3494 : Inport  Port=3
  Block3495 : Constant  Value=0  OutDataTypeStr=double  SampleTime=-1
  Block3496 : Constant  OutDataTypeStr=double  SampleTime=-1
  Block3497 : Constant  OutDataTypeStr=double  SampleTime=-1
  Block3498 : Constant  Value=0  OutDataTypeStr=double  SampleTime=-1
  Block3499 : Constant  Value=2  OutDataTypeStr=double  SampleTime=-1
  Block3500 : Constant  OutDataTypeStr=double  SampleTime=-1
  Block3501 : Constant  Value=143  OutDataTypeStr=double  SampleTime=-1
  Block3502 : Demux  Outputs=2
  Block3503 : Logic  Operator=NOT
  Block3504 : Mux  Inputs=2
  Block3505 : Mux  Inputs=2
  Block3506 : Sum  OutDataTypeStr=double  RndMeth=Zero  SaturateOnIntegerOverflow=on  InputSameDT=on
  Block3507 : Sum  OutDataTypeStr=double  RndMeth=Zero  SaturateOnIntegerOverflow=on  InputSameDT=on
  Block{3508..3511} (4 alike) : Switch  Threshold=0.5  RndMeth=Zero  SaturateOnIntegerOverflow=on  InputSameDT=on
  SubSystem1285 : Subsystem
  SubSystem1286 : Subsystem
  SubSystem1287 : Subsystem
  SubSystem1288 : Subsystem
  SubSystem1289 : Subsystem  TreatAsAtomicUnit=on
  SubSystem1298 : Subsystem  TreatAsAtomicUnit=on
  SubSystem1308 : Subsystem  TreatAsAtomicUnit=on
  SubSystem1318 : Subsystem  TreatAsAtomicUnit=on
  Block3571 : Outport
  Block3572 : Outport  Port=2
  wires:
  Block3510/1 -> Block3507/2
  Block3500/1 -> Block3507/1
  Block3492/1 -> SubSystem1285/1(In1), Block3506/2, Block3504/1
  Block3501/1 -> SubSystem1285/2(In2), Block3505/1
  Block3493/1 -> SubSystem1286/1(In1), SubSystem1287/1(In1), SubSystem1288/1(In1)
  Block3495/1 -> SubSystem1286/2(In2)
  SubSystem1286/1(Out1) -> Block3508/2, SubSystem1289/Enable
  SubSystem1289/1(Block3525) -> Block3508/1
  Block3496/1 -> SubSystem1287/2(In2)
  SubSystem1287/1(Out1) -> Block3509/2, SubSystem1298/Enable
  SubSystem1298/1(Block3540) -> Block3509/1
  Block3509/1 -> Block3508/3
  Block3507/1 -> SubSystem1289/1(Block3512), SubSystem1298/1(Block3526), SubSystem1308/1(Block3541), SubSystem1318/1(Block3556)
  Block3502/1 -> Block3571/1
  Block3502/2 -> Block3572/1
  Block3508/1 -> Block3502/1
  SubSystem1285/1(Out1) -> Block3510/2
  Block3497/1 -> Block3506/1
  Block3498/1 -> Block3505/2
  Block3505/1 -> Block3510/3
  Block3506/1 -> Block3504/2
  Block3504/1 -> Block3510/1
  Block3499/1 -> SubSystem1288/2(In2)
  SubSystem1288/1(Out1) -> Block3503/1, SubSystem1308/Enable, Block3511/2
  Block3503/1 -> SubSystem1318/Enable
  SubSystem1308/1(Block3555) -> Block3511/1
  SubSystem1318/1(Block3570) -> Block3511/3
  Block3511/1 -> Block3509/3
  Block3494/1 -> SubSystem1289/2(Block3513), SubSystem1298/2(Block3527), SubSystem1308/2(Block3542), SubSystem1318/2(Block3557)
